simulink library "R9000_FirmwareLib"  (R2024b)
settings: Solver=VariableStepAuto  StartTime=0.0  StopTime=10.0  RelTol=1e-3
libraries: mbd_s32k3_io_library x82, mbd_s32k3_isr_library x15, mbd_s32k3_communication_library x10, mbd_s32k3_utility_library x1
(part 5 of 7)

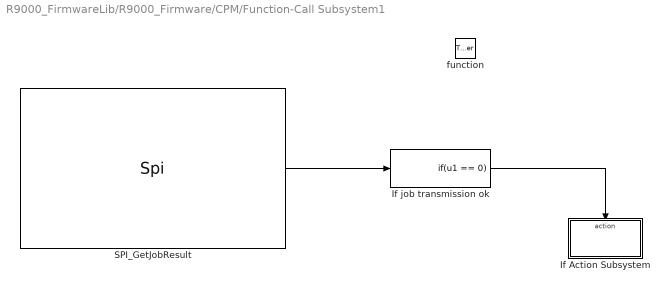
== system R9000_FirmwareLib/R9000_Firmware/CPM/Function-Call Subsystem1 ==
  function : TriggerPort  TriggerType=function-call  VariantControl=Variant
  If Action Subsystem : Subsystem  TreatAsAtomicUnit=on
  If job transmission ok : If  IfExpression=u1 == 0  ShowElse=off
  SPI_GetJobResult : mbd_s32k3_communication_library/Spi  api_func=Spi_GetJobResult  channel=SpiChannel_0  job=SpiJob_0  sequence=SpiSequence_0  hwunit=CSIB0  mode=Polling  text=|
      | CommonPublishedInformation - CommonPublishedInformation 
      | CommonPublishedInformation/ArReleaseMajorVersion - 4 
      | CommonPublishedInformation/ArReleaseMinorVersion - 4 
      | CommonPublishedInformation/ArReleaseRevisionVersion - 0 
      | CommonPublishedInformation/ModuleId - 83 
      | CommonPublishedInformation/SwMajorVersion - 2 
      | CommonPublishedInformation/SwMinorVersion - 0 
      | CommonPublishedInformation/SwPatchVersion - 0 
      | CommonPublishedInformation/VendorId - 43 
      | IMPLEMENTATION-CONFIG-VARIANT - VARIANT-POST-BUILD 
      | POST_BUILD_VARIANT_USED - true 
      | SpiAutosarExt - SpiAutosarExt 
      | SpiAutosarExt/SpiAllowBigSizeCollections - false 
      | SpiAutosarExt/SpiDisableDemReportErrorStatus - false 
      | SpiAutosarExt/SpiEnableDmaContMemTransferSupport - false 
      | SpiAutosarExt/SpiEnableDmaFastTransferSupport - false 
      | SpiAutosarExt/SpiEnableHWUnitAsyncMode - false 
      | SpiAutosarExt/SpiEnableUserModeSupport - false 
      | SpiAutosarExt/SpiFlexioEnable - false 
      | SpiAutosarExt/SpiHalfDuplexModeSupport - false 
      | SpiAutosarExt/SpiJobStartNotificationEnable - false 
      | SpiAutosarExt/SpiUseLegacyEBLength - false 
      | SpiDriver - SpiDriver 
      | SpiDriver/SpiChannel - SpiChannel_0 SpiChannel_1 
      | SpiDriver/SpiChannel_0/Index - 0 
      | SpiDriver/SpiChannel_0/SpiByteSwapTransfer - false 
      | SpiDriver/SpiChannel_0/SpiChannelId - 0 
      | SpiDriver/SpiChannel_0/SpiChannelType - IB 
      | SpiDriver/SpiChannel_0/SpiDataWidth - 32 
      | SpiDriver/SpiChannel_0/SpiEbMaxLength - 1000 
      | SpiDriver/SpiChannel_0/SpiIbNBuffers - 4 
      | SpiDriver/SpiChannel_0/SpiTransferStart - MSB 
      | SpiDriver/SpiChannel_1/Index - 1 
      | SpiDriver/SpiChannel_1/SpiByteSwapTransfer - false 
      | SpiDriver/SpiChannel_1/SpiChannelId - 1 
      | SpiDriver/SpiChannel_1/SpiChannelType - IB 
      | SpiDriver/SpiChannel_1/SpiDataWidth - 8 
      | SpiDriver/SpiChannel_1/SpiEbMaxLength - 1000 
      | SpiDriver/SpiChannel_1/SpiIbNBuffers - 1 
      | SpiDriver/SpiChannel_1/SpiTransferStart - MSB 
      | SpiDriver/SpiExternalDevice - SpiExternalDevice_0 SpiExternalDevice_1 
      | SpiDriver/SpiExternalDevice_0/Index - 0 
      | SpiDriver/SpiExternalDevice_0/SpiBaudrate - 1000000.0 
      | SpiDriver/SpiExternalDevice_0/SpiCsBehavior - CS_KEEP_ASSERTED 
      | SpiDriver/SpiExternalDevice_0/SpiCsIdentifier - PCS0 
      | SpiDriver/SpiExternalDevice_0/SpiCsPolarity - LOW 
      | SpiDriver/SpiExternalDevice_0/SpiCsSelection - CS_VIA_PERIPHERAL_ENGINE 
      | SpiDriver/SpiExternalDevice_0/SpiDataShiftEdge - LEADING 
      | SpiDriver/SpiExternalDevice_0/SpiDeviceHalfDuplexSupport - false 
      | SpiDriver/SpiExternalDevice_0/SpiEnableCs - true 
      | SpiDriver/SpiExternalDevice_0/SpiHalfDuplexPinSelect - HALF_DUPLEX_SIN 
      | SpiDriver/SpiExternalDevice_0/SpiHostRequest - DISABLE 
      | SpiDriver/SpiExternalDevice_0/SpiHwUnit - CSIB0 
      | SpiDriver/SpiExternalDevice_0/SpiShiftClockIdleLevel - LOW 
      | SpiDriver/SpiExternalDevice_0/SpiTimeClk2Cs - 2.0E-7 
      | SpiDriver/SpiExternalDevice_0/SpiTimeCs2Clk - 2.0E-7 
      | SpiDriver/SpiExternalDevice_0/SpiTimeCs2Cs - 4.0E-7 
      | SpiDriver/SpiExternalDevice_0/SpiTransferWidth - TRANSFER_1_BIT 
      | SpiDriver/SpiExternalDevice_0/SpiUseBaudrateConfig - false 
      | SpiDriver/SpiExternalDevice_1/Index - 1 
      | SpiDriver/SpiExternalDevice_1/SpiBaudrate - 500000.0 
      | SpiDriver/SpiExternalDevice_1/SpiCsBehavior - CS_KEEP_ASSERTED 
      | SpiDriver/SpiExternalDevice_1/SpiCsIdentifier - PCS3 
      | SpiDriver/SpiExternalDevice_1/SpiCsPolarity - LOW 
      | SpiDriver/SpiExternalDevice_1/SpiCsSelection - CS_VIA_PERIPHERAL_ENGINE 
      | SpiDriver/SpiExternalDevice_1/SpiDataShiftEdge - LEADING 
      | SpiDriver/SpiExternalDevice_1/SpiDeviceHalfDuplexSupport - false 
      | SpiDriver/SpiExternalDevice_1/SpiEnableCs - true 
      | SpiDriver/SpiExternalDevice_1/SpiHalfDuplexPinSelect - HALF_DUPLEX_SIN 
      | SpiDriver/SpiExternalDevice_1/SpiHostRequest - DISABLE 
      | SpiDriver/SpiExternalDevice_1/SpiHwUnit - CSIB1 
      | SpiDriver/SpiExternalDevice_1/SpiShiftClockIdleLevel - LOW 
      | SpiDriver/SpiExternalDevice_1/SpiTimeClk2Cs - 2.0E-7 
      | SpiDriver/SpiExternalDevice_1/SpiTimeCs2Clk - 2.0E-7 
      | SpiDriver/SpiExternalDevice_1/SpiTimeCs2Cs - 4.0E-7 
      | SpiDriver/SpiExternalDevice_1/SpiTransferWidth - TRANSFER_1_BIT 
      | SpiDriver/SpiExternalDevice_1/SpiUseBaudrateConfig - false 
      | SpiDriver/SpiJob - SpiJob_0 SpiJob_1 
      | SpiDriver/SpiJob_0/Index - 0 
      | SpiDriver/SpiJob_0/SpiChannelList - SpiChannelList_0 
      | SpiDriver/SpiJob_0/SpiChannelList_0/Index - 0 
      | SpiDriver/SpiJob_0/SpiChannelList_0/SpiChannelAssignment - /Spi/Spi/SpiDriver/SpiChannel_0 
      | SpiDriver/SpiJob_0/SpiChannelList_0/SpiChannelIndex - 0 
      | SpiDriver/SpiJob_0/SpiDeviceAssignment - /Spi/Spi/SpiDriver/SpiExternalDevice_0 
      | SpiDriver/SpiJob_0/SpiJobEndNotification - MBDT_SPI_end_job0_callback 
      | SpiDriver/SpiJob_0/SpiJobId - 0 
      | SpiDriver/SpiJob_0/SpiJobPriority - 0 
      | SpiDriver/SpiJob_1/Index - 1 
      | SpiDriver/SpiJob_1/SpiChannelList - SpiChannelList_0 
      | SpiDriver/SpiJob_1/SpiChannelList_0/Index - 0 
      | SpiDriver/SpiJob_1/SpiChannelList_0/SpiChannelAssignment - /Spi/Spi/SpiDriver/SpiChannel_1 
      | SpiDriver/SpiJob_1/SpiChannelList_0/SpiChannelIndex - 0 
      | SpiDriver/SpiJob_1/SpiDeviceAssignment - /Spi/Spi/SpiDriver/SpiExternalDevice_1 
      | SpiDriver/SpiJob_1/SpiJobId - 1 
      | SpiDriver/SpiJob_1/SpiJobPriority - 0 
      | SpiDriver/SpiSequence - SpiSequence_0 SpiSequence_1 
      | SpiDriver/SpiSequence_0/Index - 0 
      | SpiDriver/SpiSequence_0/SpiDmaContMemTransferSequenceEnable - false 
      | SpiDriver/SpiSequence_0/SpiEnableDmaFastTransfer - false 
      | SpiDriver/SpiSequence_0/SpiInterruptibleSequence - false 
      | SpiDriver/SpiSequence_0/SpiJobAssignment - /Spi/Spi/SpiDriver/SpiJob_0 
      | SpiDriver/SpiSequence_0/SpiSequenceId - 0 
      | SpiDriver/SpiSequence_1/Index - 1 
      | SpiDriver/SpiSequence_1/SpiDmaContMemTransferSequenceEnable - false 
      | SpiDriver/SpiSequence_1/SpiEnableDmaFastTransfer - false 
      | SpiDriver/SpiSequence_1/SpiInterruptibleSequence - false 
      | SpiDriver/SpiSequence_1/SpiJobAssignment - /Spi/Spi/SpiDriver/SpiJob_1 
      | SpiDriver/SpiSequence_1/SpiSeqEndNotification - MBDT_SPI_end_sequence1_callback 
      | SpiDriver/SpiSequence_1/SpiSequenceId - 1 
      | SpiGeneral - SpiGeneral 
      | SpiGeneral/SpiCancelApi - true 
      | SpiGeneral/SpiChannelBuffersAllowed - 2 
      | SpiGeneral/SpiDevErrorDetect - true 
      | SpiGeneral/SpiGlobalDmaEnable - false 
      | SpiGeneral/SpiHwStatusApi - true 
      | SpiGeneral/SpiInterruptibleSeqAllowed - true 
      | SpiGeneral/SpiLevelDelivered - 2 
      | SpiGeneral/SpiMultipartitionSupport - false 
      | SpiGeneral/SpiPhyUnit - SpiPhyUnit_0 SpiPhyUnit_1 
      | SpiGeneral/SpiPhyUnit_0/Index - 0 
      | SpiGeneral/SpiPhyUnit_0/SpiPhyUnitAsyncUseDma - false 
      | SpiGeneral/SpiPhyUnit_0/SpiPhyUnitClockRef - /Mcu/Mcu/McuModuleConfiguration/McuClockSettingConfig_0/SPI_CLK 
      | SpiGeneral/SpiPhyUnit_0/SpiPhyUnitMapping - LPSPI_2 
      | SpiGeneral/SpiPhyUnit_0/SpiPhyUnitMode - SPI_MASTER 
      | SpiGeneral/SpiPhyUnit_0/SpiPinConfiguration - 0 
      | SpiGeneral/SpiPhyUnit_0/SpiSamplePoint - 0 
      | SpiGeneral/SpiPhyUnit_1/Index - 1 
      | SpiGeneral/SpiPhyUnit_1/SpiPhyUnitAsyncUseDma - false 
      | SpiGeneral/SpiPhyUnit_1/SpiPhyUnitClockRef - /Mcu/Mcu/McuModuleConfiguration/McuClockSettingConfig_0/SPI_CLK 
      | SpiGeneral/SpiPhyUnit_1/SpiPhyUnitMapping - LPSPI_1 
      | SpiGeneral/SpiPhyUnit_1/SpiPhyUnitMode - SPI_SLAVE 
      | SpiGeneral/SpiPhyUnit_1/SpiPinConfiguration - 0 
      | SpiGeneral/SpiPhyUnit_1/SpiSamplePoint - 0 
      | SpiGeneral/SpiSupportConcurrentSyncTransmit - false 
      | SpiGeneral/SpiTimeoutMethod - OSIF_COUNTER_DUMMY 
      | SpiGeneral/SpiTransmitTimeout - 50000 
      | SpiGeneral/SpiVersionInfoApi - true 
      | SpiPublishedInformation - SpiPublishedInformation 
      | SpiPublishedInformation/SpiMaxHwUnit - 0
      settings: SourceProductName=Communication Blocks
  wires:
  SPI_GetJobResult/1 -> If job transmission ok/1
  If job transmission ok/1 -> If Action Subsystem/Action

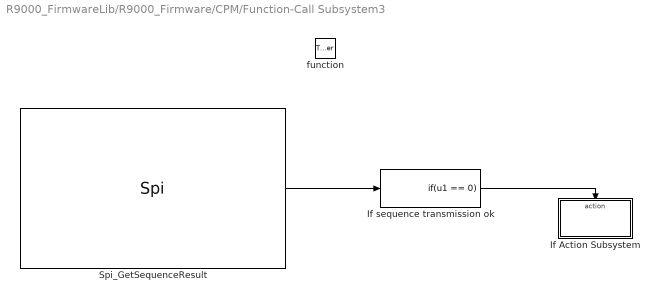
== system R9000_FirmwareLib/R9000_Firmware/CPM/Function-Call Subsystem3 ==
  function : TriggerPort  TriggerType=function-call  VariantControl=Variant
  If Action Subsystem : Subsystem  TreatAsAtomicUnit=on
  If sequence transmission ok : If  IfExpression=u1 == 0  ShowElse=off
  Spi_GetSequenceResult : mbd_s32k3_communication_library/Spi  Priority=3  api_func=Spi_GetSequenceResult  channel=SpiChannel_0  job=SpiJob_0  sequence=SpiSequence_1  hwunit=CSIB0  mode=Polling  text=|
      | CommonPublishedInformation - CommonPublishedInformation 
      | CommonPublishedInformation/ArReleaseMajorVersion - 4 
      | CommonPublishedInformation/ArReleaseMinorVersion - 4 
      | CommonPublishedInformation/ArReleaseRevisionVersion - 0 
      | CommonPublishedInformation/ModuleId - 83 
      | CommonPublishedInformation/SwMajorVersion - 2 
      | CommonPublishedInformation/SwMinorVersion - 0 
      | CommonPublishedInformation/SwPatchVersion - 0 
      | CommonPublishedInformation/VendorId - 43 
      | IMPLEMENTATION-CONFIG-VARIANT - VARIANT-POST-BUILD 
      | POST_BUILD_VARIANT_USED - true 
      | SpiAutosarExt - SpiAutosarExt 
      | SpiAutosarExt/SpiAllowBigSizeCollections - false 
      | SpiAutosarExt/SpiDisableDemReportErrorStatus - false 
      | SpiAutosarExt/SpiEnableDmaContMemTransferSupport - false 
      | SpiAutosarExt/SpiEnableDmaFastTransferSupport - false 
      | SpiAutosarExt/SpiEnableHWUnitAsyncMode - false 
      | SpiAutosarExt/SpiEnableUserModeSupport - false 
      | SpiAutosarExt/SpiFlexioEnable - false 
      | SpiAutosarExt/SpiHalfDuplexModeSupport - false 
      | SpiAutosarExt/SpiJobStartNotificationEnable - false 
      | SpiAutosarExt/SpiUseLegacyEBLength - false 
      | SpiDriver - SpiDriver 
      | SpiDriver/SpiChannel - SpiChannel_0 SpiChannel_1 
      | SpiDriver/SpiChannel_0/Index - 0 
      | SpiDriver/SpiChannel_0/SpiByteSwapTransfer - false 
      | SpiDriver/SpiChannel_0/SpiChannelId - 0 
      | SpiDriver/SpiChannel_0/SpiChannelType - IB 
      | SpiDriver/SpiChannel_0/SpiDataWidth - 32 
      | SpiDriver/SpiChannel_0/SpiEbMaxLength - 1000 
      | SpiDriver/SpiChannel_0/SpiIbNBuffers - 4 
      | SpiDriver/SpiChannel_0/SpiTransferStart - MSB 
      | SpiDriver/SpiChannel_1/Index - 1 
      | SpiDriver/SpiChannel_1/SpiByteSwapTransfer - false 
      | SpiDriver/SpiChannel_1/SpiChannelId - 1 
      | SpiDriver/SpiChannel_1/SpiChannelType - IB 
      | SpiDriver/SpiChannel_1/SpiDataWidth - 8 
      | SpiDriver/SpiChannel_1/SpiEbMaxLength - 1000 
      | SpiDriver/SpiChannel_1/SpiIbNBuffers - 1 
      | SpiDriver/SpiChannel_1/SpiTransferStart - MSB 
      | SpiDriver/SpiExternalDevice - SpiExternalDevice_0 SpiExternalDevice_1 
      | SpiDriver/SpiExternalDevice_0/Index - 0 
      | SpiDriver/SpiExternalDevice_0/SpiBaudrate - 1000000.0 
      | SpiDriver/SpiExternalDevice_0/SpiCsBehavior - CS_KEEP_ASSERTED 
      | SpiDriver/SpiExternalDevice_0/SpiCsIdentifier - PCS0 
      | SpiDriver/SpiExternalDevice_0/SpiCsPolarity - LOW 
      | SpiDriver/SpiExternalDevice_0/SpiCsSelection - CS_VIA_PERIPHERAL_ENGINE 
      | SpiDriver/SpiExternalDevice_0/SpiDataShiftEdge - LEADING 
      | SpiDriver/SpiExternalDevice_0/SpiDeviceHalfDuplexSupport - false 
      | SpiDriver/SpiExternalDevice_0/SpiEnableCs - true 
      | SpiDriver/SpiExternalDevice_0/SpiHalfDuplexPinSelect - HALF_DUPLEX_SIN 
      | SpiDriver/SpiExternalDevice_0/SpiHostRequest - DISABLE 
      | SpiDriver/SpiExternalDevice_0/SpiHwUnit - CSIB0 
      | SpiDriver/SpiExternalDevice_0/SpiShiftClockIdleLevel - LOW 
      | SpiDriver/SpiExternalDevice_0/SpiTimeClk2Cs - 2.0E-7 
      | SpiDriver/SpiExternalDevice_0/SpiTimeCs2Clk - 2.0E-7 
      | SpiDriver/SpiExternalDevice_0/SpiTimeCs2Cs - 4.0E-7 
      | SpiDriver/SpiExternalDevice_0/SpiTransferWidth - TRANSFER_1_BIT 
      | SpiDriver/SpiExternalDevice_0/SpiUseBaudrateConfig - false 
      | SpiDriver/SpiExternalDevice_1/Index - 1 
      | SpiDriver/SpiExternalDevice_1/SpiBaudrate - 500000.0 
      | SpiDriver/SpiExternalDevice_1/SpiCsBehavior - CS_KEEP_ASSERTED 
      | SpiDriver/SpiExternalDevice_1/SpiCsIdentifier - PCS3 
      | SpiDriver/SpiExternalDevice_1/SpiCsPolarity - LOW 
      | SpiDriver/SpiExternalDevice_1/SpiCsSelection - CS_VIA_PERIPHERAL_ENGINE 
      | SpiDriver/SpiExternalDevice_1/SpiDataShiftEdge - LEADING 
      | SpiDriver/SpiExternalDevice_1/SpiDeviceHalfDuplexSupport - false 
      | SpiDriver/SpiExternalDevice_1/SpiEnableCs - true 
      | SpiDriver/SpiExternalDevice_1/SpiHalfDuplexPinSelect - HALF_DUPLEX_SIN 
      | SpiDriver/SpiExternalDevice_1/SpiHostRequest - DISABLE 
      | SpiDriver/SpiExternalDevice_1/SpiHwUnit - CSIB1 
      | SpiDriver/SpiExternalDevice_1/SpiShiftClockIdleLevel - LOW 
      | SpiDriver/SpiExternalDevice_1/SpiTimeClk2Cs - 2.0E-7 
      | SpiDriver/SpiExternalDevice_1/SpiTimeCs2Clk - 2.0E-7 
      | SpiDriver/SpiExternalDevice_1/SpiTimeCs2Cs - 4.0E-7 
      | SpiDriver/SpiExternalDevice_1/SpiTransferWidth - TRANSFER_1_BIT 
      | SpiDriver/SpiExternalDevice_1/SpiUseBaudrateConfig - false 
      | SpiDriver/SpiJob - SpiJob_0 SpiJob_1 
      | SpiDriver/SpiJob_0/Index - 0 
      | SpiDriver/SpiJob_0/SpiChannelList - SpiChannelList_0 
      | SpiDriver/SpiJob_0/SpiChannelList_0/Index - 0 
      | SpiDriver/SpiJob_0/SpiChannelList_0/SpiChannelAssignment - /Spi/Spi/SpiDriver/SpiChannel_0 
      | SpiDriver/SpiJob_0/SpiChannelList_0/SpiChannelIndex - 0 
      | SpiDriver/SpiJob_0/SpiDeviceAssignment - /Spi/Spi/SpiDriver/SpiExternalDevice_0 
      | SpiDriver/SpiJob_0/SpiJobEndNotification - MBDT_SPI_end_job0_callback 
      | SpiDriver/SpiJob_0/SpiJobId - 0 
      | SpiDriver/SpiJob_0/SpiJobPriority - 0 
      | SpiDriver/SpiJob_1/Index - 1 
      | SpiDriver/SpiJob_1/SpiChannelList - SpiChannelList_0 
      | SpiDriver/SpiJob_1/SpiChannelList_0/Index - 0 
      | SpiDriver/SpiJob_1/SpiChannelList_0/SpiChannelAssignment - /Spi/Spi/SpiDriver/SpiChannel_1 
      | SpiDriver/SpiJob_1/SpiChannelList_0/SpiChannelIndex - 0 
      | SpiDriver/SpiJob_1/SpiDeviceAssignment - /Spi/Spi/SpiDriver/SpiExternalDevice_1 
      | SpiDriver/SpiJob_1/SpiJobId - 1 
      | SpiDriver/SpiJob_1/SpiJobPriority - 0 
      | SpiDriver/SpiSequence - SpiSequence_0 SpiSequence_1 
      | SpiDriver/SpiSequence_0/Index - 0 
      | SpiDriver/SpiSequence_0/SpiDmaContMemTransferSequenceEnable - false 
      | SpiDriver/SpiSequence_0/SpiEnableDmaFastTransfer - false 
      | SpiDriver/SpiSequence_0/SpiInterruptibleSequence - false 
      | SpiDriver/SpiSequence_0/SpiJobAssignment - /Spi/Spi/SpiDriver/SpiJob_0 
      | SpiDriver/SpiSequence_0/SpiSequenceId - 0 
      | SpiDriver/SpiSequence_1/Index - 1 
      | SpiDriver/SpiSequence_1/SpiDmaContMemTransferSequenceEnable - false 
      | SpiDriver/SpiSequence_1/SpiEnableDmaFastTransfer - false 
      | SpiDriver/SpiSequence_1/SpiInterruptibleSequence - false 
      | SpiDriver/SpiSequence_1/SpiJobAssignment - /Spi/Spi/SpiDriver/SpiJob_1 
      | SpiDriver/SpiSequence_1/SpiSeqEndNotification - MBDT_SPI_end_sequence1_callback 
      | SpiDriver/SpiSequence_1/SpiSequenceId - 1 
      | SpiGeneral - SpiGeneral 
      | SpiGeneral/SpiCancelApi - true 
      | SpiGeneral/SpiChannelBuffersAllowed - 2 
      | SpiGeneral/SpiDevErrorDetect - true 
      | SpiGeneral/SpiGlobalDmaEnable - false 
      | SpiGeneral/SpiHwStatusApi - true 
      | SpiGeneral/SpiInterruptibleSeqAllowed - true 
      | SpiGeneral/SpiLevelDelivered - 2 
      | SpiGeneral/SpiMultipartitionSupport - false 
      | SpiGeneral/SpiPhyUnit - SpiPhyUnit_0 SpiPhyUnit_1 
      | SpiGeneral/SpiPhyUnit_0/Index - 0 
      | SpiGeneral/SpiPhyUnit_0/SpiPhyUnitAsyncUseDma - false 
      | SpiGeneral/SpiPhyUnit_0/SpiPhyUnitClockRef - /Mcu/Mcu/McuModuleConfiguration/McuClockSettingConfig_0/SPI_CLK 
      | SpiGeneral/SpiPhyUnit_0/SpiPhyUnitMapping - LPSPI_2 
      | SpiGeneral/SpiPhyUnit_0/SpiPhyUnitMode - SPI_MASTER 
      | SpiGeneral/SpiPhyUnit_0/SpiPinConfiguration - 0 
      | SpiGeneral/SpiPhyUnit_0/SpiSamplePoint - 0 
      | SpiGeneral/SpiPhyUnit_1/Index - 1 
      | SpiGeneral/SpiPhyUnit_1/SpiPhyUnitAsyncUseDma - false 
      | SpiGeneral/SpiPhyUnit_1/SpiPhyUnitClockRef - /Mcu/Mcu/McuModuleConfiguration/McuClockSettingConfig_0/SPI_CLK 
      | SpiGeneral/SpiPhyUnit_1/SpiPhyUnitMapping - LPSPI_1 
      | SpiGeneral/SpiPhyUnit_1/SpiPhyUnitMode - SPI_SLAVE 
      | SpiGeneral/SpiPhyUnit_1/SpiPinConfiguration - 0 
      | SpiGeneral/SpiPhyUnit_1/SpiSamplePoint - 0 
      | SpiGeneral/SpiSupportConcurrentSyncTransmit - false 
      | SpiGeneral/SpiTimeoutMethod - OSIF_COUNTER_DUMMY 
      | SpiGeneral/SpiTransmitTimeout - 50000 
      | SpiGeneral/SpiVersionInfoApi - true 
      | SpiPublishedInformation - SpiPublishedInformation 
      | SpiPublishedInformation/SpiMaxHwUnit - 0
      settings: SourceProductName=Communication Blocks
  wires:
  Spi_GetSequenceResult/1 -> If sequence transmission ok/1
  If sequence transmission ok/1 -> If Action Subsystem/Action
